simulink model "TosAeb"  (R2021a)
settings: Solver=FixedStepDiscrete  StartTime=0.0  StopTime=inf  FixedStep=0.01  RelTol=1e-3
undefined symbols (not defined in the file or in its folder): ACCModSts, AccSts1, AEB_ObjectMovingOrientation, AEB_ObjectMovingState, CN_BTCActive, CN_CurrentGearR, CN_CurrentGearR_DHT, CN_PTCActive, CN_VDCActive, CN_VSC_EspOff, CN_VSC_FCW_AEB_Error, CN_VSC_FCW_AEB_NoDispay, CN_VSC_FCW_AEB_PerfmDgdtn, CN_VSC_FCW_AEB_TempUnAvlbl, CN_VSC_VehStandstill, CNaccelAvoidMax, CNaccelAvoidMin, CNbrakePedalPress, CNcomfortStrLeftAccelMax, CNcomfortStrLeftAccelMin, CNcomfortStrRightAccelMax, CNcomfortStrRightAccelMin, CNDrvDoorOpen, CNDrvModeEco, CNDrvModeNorm, CNDrvModeOther, CNDrvModeSport, CNDrvSBRreminder, CNNull, CNobjInvalidID, CNPassDoorOpen, CNPassSBRreminder, CNPEBSAccelNegInf, CNPEBSAccelNull, CNPEBSTimeInf, CNPEBSTimeNull, CNSTOPMAX, CNSTOPNULL, CNsubjWidth, CNSwtOFF, CNSwtON, CNTTCMAX, CNTTCNULL, CNVehConfigAEBCycNone, CNVehConfigAEBNone, CNVehConfigAEBPedNone, CNVehConfigFCWNone, CNVehConfigJANone, CollisionSeverity, FALSE, FCWSnvty, JACycObjCalcInfoDefaultParams, JAPedObjCalcInfoDefaultParams, JAVehObjCalcInfoDefaultParams, latCycObjCalcInfoDefaultParams, latPedObjCalcInfoDefaultParams, longObjCalcInfoDefaultParams, ModSts, movingStatus, ObjectType ... (+1112)
libraries: simulink x3031, AEBSFunctionLib x50, Ta_Library x33, AEBSStateMachineLib x23, Mylibrary x12, simulink_extras x7, ObjSelect_lib x3, dspstat3 x2
(part 101 of 102)

== stateflow chart TosAeb/ELK_Objsel/Target_Selection/If Action Subsystem/ELK_TargetSelection/If Action Threat_Assessment_Left/Trigger Hold1/Chart ==
  (default) -> (junction 1)
  (junction 1) -> (junction 2) [order 1] : [(Trigger==1)]...
      | {count = 0;}
  (junction 1) -> (junction 12) [order 2]
  (junction 2) -> (junction 10)
  (junction 12) -> (junction 10) [order 1] : [(count<= hold_time)&&(count>0)]
  (junction 12) -> (junction 3) [order 2] : {Out = 0;}
  (junction 10) -> (junction 15) : {
      | Out = 1;...
      | count = count + 10;
      | }
  (junction 3) -> (junction 15)
  (junction 15) -> (junction 8)
  data: Trigger input; hold_time input; Out output double; count local double

== stateflow chart TosAeb/ELK_Objsel/Target_Selection/If Action Subsystem/ELK_TargetSelection/If Action Threat_Assessment_Left1/Delay_Trig/Chart ==
  state OFF
    en: Delay_Trig =0;
    count= 0;
    settings: description=_GWMH9P4.LKAFN.111_17_
  state ON
    en: Delay_Trig = 0;
    count++;
    du:count++;
    settings: description=_GWMH9P4.LKAFN.111_18_
  state Trig
    en: Delay_Trig = 1;
    settings: description=_GWMH9P4.LKAFN.111_19_
  (default) -> OFF
  OFF -> ON : [(Input)]
  ON -> OFF [order 2] : [!Input]
  Trig -> OFF : [!Input]
  ON -> Trig [order 1] : [(Input) && (count>= Delay_Trig_count)]
  data: Input input; Delay_Trig_count input; Delay_Trig output double; count local double

== stateflow chart TosAeb/ELK_Objsel/Target_Selection/If Action Subsystem/ELK_TargetSelection/If Action Threat_Assessment_Left1/Delay_Trig1/Chart ==
  state OFF
    en: Delay_Trig =0;
    count= 0;
    settings: description=_GWMH9P4.LKAFN.111_17_
  state ON
    en: Delay_Trig = 0;
    count++;
    du:count++;
    settings: description=_GWMH9P4.LKAFN.111_18_
  state Trig
    en: Delay_Trig = 1;
    settings: description=_GWMH9P4.LKAFN.111_19_
  (default) -> OFF
  OFF -> ON : [(Input)]
  ON -> OFF [order 2] : [!Input]
  Trig -> OFF : [!Input]
  ON -> Trig [order 1] : [(Input) && (count>= Delay_Trig_count)]
  data: Input input; Delay_Trig_count input; Delay_Trig output double; count local double

== stateflow chart TosAeb/ELK_Objsel/Target_Selection/If Action Subsystem/ELK_TargetSelection/If Action Threat_Assessment_Left1/Hysteresis ==
  state Flag_False
    en:
    Flag = 0;
    du:
    Flag = 0;
    settings: description=_GWMH9P5.ELKFN.111_3_
  state Flag_True
    en:
    Flag = 1;
    du:
    Flag = 1;
    settings: description=_GWMH9P5.ELKFN.111_4_
  (default) -> Flag_False
  Flag_False -> Flag_True : [Input_1 == 1]
  Flag_True -> Flag_False : [Input_2 == 1]
  data: Input_1 input; Input_2 input; Flag output double

== stateflow chart TosAeb/ELK_Objsel/Target_Selection/If Action Subsystem/ELK_TargetSelection/If Action Threat_Assessment_Left1/Hysteresis1 ==
  state Flag_False
    en:
    Flag = 0;
    du:
    Flag = 0;
    settings: description=_GWMH9P5.ELKFN.111_3_
  state Flag_True
    en:
    Flag = 1;
    du:
    Flag = 1;
    settings: description=_GWMH9P5.ELKFN.111_4_
  (default) -> Flag_False
  Flag_False -> Flag_True : [Input_1 == 1]
  Flag_True -> Flag_False : [Input_2 == 1]
  data: Input_1 input; Input_2 input; Flag output double

== stateflow chart TosAeb/ELK_Objsel/Target_Selection/If Action Subsystem/ELK_TargetSelection/If Action Threat_Assessment_Left1/Hysteresis2 ==
  state Flag_False
    en:
    Flag = 0;
    du:
    Flag = 0;
    settings: description=_GWMH9P5.ELKFN.111_3_
  state Flag_True
    en:
    Flag = 1;
    du:
    Flag = 1;
    settings: description=_GWMH9P5.ELKFN.111_4_
  (default) -> Flag_False
  Flag_False -> Flag_True : [Input_1 == 1]
  Flag_True -> Flag_False : [Input_2 == 1]
  data: Input_1 input; Input_2 input; Flag output double

== stateflow chart TosAeb/ELK_Objsel/Target_Selection/If Action Subsystem/ELK_TargetSelection/If Action Threat_Assessment_Left1/Trigger Hold/Chart ==
  (default) -> (junction 1)
  (junction 1) -> (junction 2) [order 1] : [(Trigger==1)]...
      | {count = 0;}
  (junction 1) -> (junction 12) [order 2]
  (junction 2) -> (junction 10)
  (junction 12) -> (junction 10) [order 1] : [(count<= hold_time)&&(count>0)]
  (junction 12) -> (junction 3) [order 2] : {Out = 0;}
  (junction 10) -> (junction 15) : {
      | Out = 1;...
      | count = count + 10;
      | }
  (junction 3) -> (junction 15)
  (junction 15) -> (junction 8)
  data: Trigger input; hold_time input; Out output double; count local double

== stateflow chart TosAeb/FcwAebJa_Step_sys/ALG_AEBSystem/ALG_AEBController/AEB_LatObjInfoCalcApp/LatPedObjCalc/Subsystem/latPedObjInfoCalc/ObjColiisionRiskProbCalc/CollisionRiskProbCalc/CollisionRiskProbCalc/dyOverlapFlow ==
  (default) -> (junction 32) : {
      | 	dyOverlapLimit = dyReginObjLLCorr- dyReginObjRUCorr;
      | 	dyOverlapLimit = max(dyOverlapLimit,0.1);
      | }
  (junction 32) -> (junction 34) [order 1] : [dyReginObjLLCorr < dyReginEgoRUCorr || ...
      | dyReginObjRUCorr > dyReginEgoLLCorr]
  (junction 32) -> (junction 2) [order 2]
  (junction 34) -> (junction 36) : {
      | 	dyOverlap = 0;
      | }
  (junction 2) -> (junction 3) [order 1] : [dyReginEgoLLCorr - dyReginEgoRUCorr < dyOverlapLimit && ...
      | dyReginEgoLLCorr <= dyReginObjLLCorr &&...
      | dyReginEgoRUCorr >= dyReginObjRUCorr]
  (junction 2) -> (junction 7) [order 2]
  (junction 3) -> (junction 5) : {
      | 	dyOverlap = dyReginEgoLLCorr - dyReginEgoRUCorr;
      | }
  (junction 7) -> (junction 9) [order 1] : [dyReginObjLLCorr - dyReginEgoRUCorr < dyOverlapLimit &&...
      | dyReginObjLLCorr - dyReginEgoRUCorr >0 && ...
      | dyReginEgoLLCorr > dyReginObjLLCorr]
  (junction 7) -> (junction 13) [order 2]
  (junction 9) -> (junction 11) : {
      | 	dyOverlap = dyReginObjLLCorr - dyReginEgoRUCorr;
      | }
  (junction 13) -> (junction 15) [order 1] : [dyReginEgoLLCorr - dyReginObjRUCorr < dyOverlapLimit && ...
      | dyReginEgoLLCorr - dyReginObjRUCorr > 0 && ...
      | dyReginEgoRUCorr < dyReginObjRUCorr]
  (junction 13) -> (junction 19) [order 2] : {
      | 	dyOverlap= dyOverlapLimit;
      | }
  (junction 15) -> (junction 17) : {
      | 	dyOverlap = dyReginEgoLLCorr - dyReginObjRUCorr;
      | }
  (junction 17) -> (junction 19)
  (junction 11) -> (junction 17)
  (junction 5) -> (junction 11)
  (junction 36) -> (junction 5)
  (junction 19) -> (junction 22)
  data: dyReginEgoRUCorr input single; dyReginEgoLLCorr input single; dyReginObjRUCorr input single; dyReginObjLLCorr input single; dyOverlap output single; dyOverlapLimit output single

== stateflow chart TosAeb/ELK_Objsel/Target_Selection/If Action Subsystem/ELK_TargetSelection/If Action Threat_Assessment_Left1/Trigger Hold1/Chart ==
  (default) -> (junction 1)
  (junction 1) -> (junction 2) [order 1] : [(Trigger==1)]...
      | {count = 0;}
  (junction 1) -> (junction 12) [order 2]
  (junction 2) -> (junction 10)
  (junction 12) -> (junction 10) [order 1] : [(count<= hold_time)&&(count>0)]
  (junction 12) -> (junction 3) [order 2] : {Out = 0;}
  (junction 10) -> (junction 15) : {
      | Out = 1;...
      | count = count + 10;
      | }
  (junction 3) -> (junction 15)
  (junction 15) -> (junction 8)
  data: Trigger input; hold_time input; Out output double; count local double

== stateflow chart TosAeb/ELK_Objsel/Target_Selection/If Action Subsystem/ELK_TargetSelection/If Action Threat_Assessment_Right/Delay_Trig/Chart ==
  state OFF
    en: Delay_Trig =0;
    count= 0;
    settings: description=_GWMH9P4.LKAFN.111_17_
  state ON
    en: Delay_Trig = 0;
    count++;
    du:count++;
    settings: description=_GWMH9P4.LKAFN.111_18_
  state Trig
    en: Delay_Trig = 1;
    settings: description=_GWMH9P4.LKAFN.111_19_
  (default) -> OFF
  OFF -> ON : [(Input)]
  ON -> OFF [order 2] : [!Input]
  Trig -> OFF : [!Input]
  ON -> Trig [order 1] : [(Input) && (count>= Delay_Trig_count)]
  data: Input input; Delay_Trig_count input; Delay_Trig output double; count local double

== stateflow chart TosAeb/ELK_Objsel/Target_Selection/If Action Subsystem/ELK_TargetSelection/If Action Threat_Assessment_Right/Delay_Trig1/Chart ==
  state OFF
    en: Delay_Trig =0;
    count= 0;
    settings: description=_GWMH9P4.LKAFN.111_17_
  state ON
    en: Delay_Trig = 0;
    count++;
    du:count++;
    settings: description=_GWMH9P4.LKAFN.111_18_
  state Trig
    en: Delay_Trig = 1;
    settings: description=_GWMH9P4.LKAFN.111_19_
  (default) -> OFF
  OFF -> ON : [(Input)]
  ON -> OFF [order 2] : [!Input]
  Trig -> OFF : [!Input]
  ON -> Trig [order 1] : [(Input) && (count>= Delay_Trig_count)]
  data: Input input; Delay_Trig_count input; Delay_Trig output double; count local double

== stateflow chart TosAeb/ELK_Objsel/Target_Selection/If Action Subsystem/ELK_TargetSelection/If Action Threat_Assessment_Right/Hysteresis ==
  state Flag_False
    en:
    Flag = 0;
    du:
    Flag = 0;
    settings: description=_GWMH9P5.ELKFN.111_3_
  state Flag_True
    en:
    Flag = 1;
    du:
    Flag = 1;
    settings: description=_GWMH9P5.ELKFN.111_4_
  (default) -> Flag_False
  Flag_False -> Flag_True : [Input_1 == 1]
  Flag_True -> Flag_False : [Input_2 == 1]
  data: Input_1 input; Input_2 input; Flag output double

== stateflow chart TosAeb/ELK_Objsel/Target_Selection/If Action Subsystem/ELK_TargetSelection/If Action Threat_Assessment_Right/Hysteresis1 ==
  state Flag_False
    en:
    Flag = 0;
    du:
    Flag = 0;
    settings: description=_GWMH9P5.ELKFN.111_3_
  state Flag_True
    en:
    Flag = 1;
    du:
    Flag = 1;
    settings: description=_GWMH9P5.ELKFN.111_4_
  (default) -> Flag_False
  Flag_False -> Flag_True : [Input_1 == 1]
  Flag_True -> Flag_False : [Input_2 == 1]
  data: Input_1 input; Input_2 input; Flag output double

== stateflow chart TosAeb/ELK_Objsel/Target_Selection/If Action Subsystem/ELK_TargetSelection/If Action Threat_Assessment_Right/Hysteresis2 ==
  state Flag_False
    en:
    Flag = 0;
    du:
    Flag = 0;
    settings: description=_GWMH9P5.ELKFN.111_3_
  state Flag_True
    en:
    Flag = 1;
    du:
    Flag = 1;
    settings: description=_GWMH9P5.ELKFN.111_4_
  (default) -> Flag_False
  Flag_False -> Flag_True : [Input_1 == 1]
  Flag_True -> Flag_False : [Input_2 == 1]
  data: Input_1 input; Input_2 input; Flag output double

== stateflow chart TosAeb/ELK_Objsel/Target_Selection/If Action Subsystem/ELK_TargetSelection/If Action Threat_Assessment_Right/Trigger Hold/Chart ==
  (default) -> (junction 1)
  (junction 1) -> (junction 2) [order 1] : [(Trigger==1)]...
      | {count = 0;}
  (junction 1) -> (junction 12) [order 2]
  (junction 2) -> (junction 10)
  (junction 12) -> (junction 10) [order 1] : [(count<= hold_time)&&(count>0)]
  (junction 12) -> (junction 3) [order 2] : {Out = 0;}
  (junction 10) -> (junction 15) : {
      | Out = 1;...
      | count = count + 10;
      | }
  (junction 3) -> (junction 15)
  (junction 15) -> (junction 8)
  data: Trigger input; hold_time input; Out output double; count local double

== stateflow chart TosAeb/ELK_Objsel/Target_Selection/If Action Subsystem/ELK_TargetSelection/If Action Threat_Assessment_Right/Trigger Hold1/Chart ==
  (default) -> (junction 1)
  (junction 1) -> (junction 2) [order 1] : [(Trigger==1)]...
      | {count = 0;}
  (junction 1) -> (junction 12) [order 2]
  (junction 2) -> (junction 10)
  (junction 12) -> (junction 10) [order 1] : [(count<= hold_time)&&(count>0)]
  (junction 12) -> (junction 3) [order 2] : {Out = 0;}
  (junction 10) -> (junction 15) : {
      | Out = 1;...
      | count = count + 10;
      | }
  (junction 3) -> (junction 15)
  (junction 15) -> (junction 8)
  data: Trigger input; hold_time input; Out output double; count local double

== stateflow chart TosAeb/ELK_Objsel/Target_Selection/If Action Subsystem/ELK_TargetSelection/If Action Threat_Assessment_Right1/Delay_Trig/Chart ==
  state OFF
    en: Delay_Trig =0;
    count= 0;
    settings: description=_GWMH9P4.LKAFN.111_17_
  state ON
    en: Delay_Trig = 0;
    count++;
    du:count++;
    settings: description=_GWMH9P4.LKAFN.111_18_
  state Trig
    en: Delay_Trig = 1;
    settings: description=_GWMH9P4.LKAFN.111_19_
  (default) -> OFF
  OFF -> ON : [(Input)]
  ON -> OFF [order 2] : [!Input]
  Trig -> OFF : [!Input]
  ON -> Trig [order 1] : [(Input) && (count>= Delay_Trig_count)]
  data: Input input; Delay_Trig_count input; Delay_Trig output double; count local double

== stateflow chart TosAeb/ELK_Objsel/Target_Selection/If Action Subsystem/ELK_TargetSelection/If Action Threat_Assessment_Right1/Delay_Trig1/Chart ==
  state OFF
    en: Delay_Trig =0;
    count= 0;
    settings: description=_GWMH9P4.LKAFN.111_17_
  state ON
    en: Delay_Trig = 0;
    count++;
    du:count++;
    settings: description=_GWMH9P4.LKAFN.111_18_
  state Trig
    en: Delay_Trig = 1;
    settings: description=_GWMH9P4.LKAFN.111_19_
  (default) -> OFF
  OFF -> ON : [(Input)]
  ON -> OFF [order 2] : [!Input]
  Trig -> OFF : [!Input]
  ON -> Trig [order 1] : [(Input) && (count>= Delay_Trig_count)]
  data: Input input; Delay_Trig_count input; Delay_Trig output double; count local double

== stateflow chart TosAeb/ELK_Objsel/Target_Selection/If Action Subsystem/ELK_TargetSelection/If Action Threat_Assessment_Right1/Hysteresis ==
  state Flag_False
    en:
    Flag = 0;
    du:
    Flag = 0;
    settings: description=_GWMH9P5.ELKFN.111_3_
  state Flag_True
    en:
    Flag = 1;
    du:
    Flag = 1;
    settings: description=_GWMH9P5.ELKFN.111_4_
  (default) -> Flag_False
  Flag_False -> Flag_True : [Input_1 == 1]
  Flag_True -> Flag_False : [Input_2 == 1]
  data: Input_1 input; Input_2 input; Flag output double

== stateflow chart TosAeb/ELK_Objsel/Target_Selection/If Action Subsystem/ELK_TargetSelection/If Action Threat_Assessment_Right1/Hysteresis1 ==
  state Flag_False
    en:
    Flag = 0;
    du:
    Flag = 0;
    settings: description=_GWMH9P5.ELKFN.111_3_
  state Flag_True
    en:
    Flag = 1;
    du:
    Flag = 1;
    settings: description=_GWMH9P5.ELKFN.111_4_
  (default) -> Flag_False
  Flag_False -> Flag_True : [Input_1 == 1]
  Flag_True -> Flag_False : [Input_2 == 1]
  data: Input_1 input; Input_2 input; Flag output double

== stateflow chart TosAeb/ELK_Objsel/Target_Selection/If Action Subsystem/ELK_TargetSelection/If Action Threat_Assessment_Right1/Hysteresis2 ==
  state Flag_False
    en:
    Flag = 0;
    du:
    Flag = 0;
    settings: description=_GWMH9P5.ELKFN.111_3_
  state Flag_True
    en:
    Flag = 1;
    du:
    Flag = 1;
    settings: description=_GWMH9P5.ELKFN.111_4_
  (default) -> Flag_False
  Flag_False -> Flag_True : [Input_1 == 1]
  Flag_True -> Flag_False : [Input_2 == 1]
  data: Input_1 input; Input_2 input; Flag output double

== stateflow chart TosAeb/ELK_Objsel/Target_Selection/If Action Subsystem/ELK_TargetSelection/If Action Threat_Assessment_Right1/Trigger Hold/Chart ==
  (default) -> (junction 1)
  (junction 1) -> (junction 2) [order 1] : [(Trigger==1)]...
      | {count = 0;}
  (junction 1) -> (junction 12) [order 2]
  (junction 2) -> (junction 10)
  (junction 12) -> (junction 10) [order 1] : [(count<= hold_time)&&(count>0)]
  (junction 12) -> (junction 3) [order 2] : {Out = 0;}
  (junction 10) -> (junction 15) : {
      | Out = 1;...
      | count = count + 10;
      | }
  (junction 3) -> (junction 15)
  (junction 15) -> (junction 8)
  data: Trigger input; hold_time input; Out output double; count local double

== stateflow chart TosAeb/ELK_Objsel/Target_Selection/If Action Subsystem/ELK_TargetSelection/If Action Threat_Assessment_Right1/Trigger Hold1/Chart ==
  (default) -> (junction 1)
  (junction 1) -> (junction 2) [order 1] : [(Trigger==1)]...
      | {count = 0;}
  (junction 1) -> (junction 12) [order 2]
  (junction 2) -> (junction 10)
  (junction 12) -> (junction 10) [order 1] : [(count<= hold_time)&&(count>0)]
  (junction 12) -> (junction 3) [order 2] : {Out = 0;}
  (junction 10) -> (junction 15) : {
      | Out = 1;...
      | count = count + 10;
      | }
  (junction 3) -> (junction 15)
  (junction 15) -> (junction 8)
  data: Trigger input; hold_time input; Out output double; count local double

== matlab function TosAeb/ELK_Objsel/Target_Selection/If Action Subsystem/MATLAB Function ==
  data: dxv_f input; vxvAbs_f output; dyv_f input; vxvRel_f input; vyvRel_f input; vxvEgo_f input; psiDtEgo_f input; vyvAbs_f output; vrAbs_f output
function [vxvAbs_f, vyvAbs_f, vrAbs_f] = fcn(dxv_f, dyv_f, vxvRel_f, vyvRel_f, vxvEgo_f, psiDtEgo_f)

if (dxv_f ~= 0)
   t_radObjAngleToRear_f = atan(dyv_f / dxv_f);
else
   t_radObjAngleToRear_f = single(0.0);
end

t_dvRadial_f = sqrt(dxv_f * dxv_f + dyv_f * dyv_f);

t_vvRadial_f = t_dvRadial_f * psiDtEgo_f;

t_sinObjAngle_f = sin(t_radObjAngleToRear_f);
t_cosObjAngle_f = cos(t_radObjAngleToRear_f);

t_tempvx_f = (vxvRel_f * t_cosObjAngle_f) + vyvRel_f * t_sinObjAngle_f;
t_tempvy_f = (vxvRel_f * (-t_sinObjAngle_f)) + vyvRel_f * t_cosObjAngle_f;

t_tempvy_f = t_tempvy_f + t_vvRadial_f;
if dxv_f <single(0)
    vxvAbs_f = (-1)*((t_tempvx_f * t_cosObjAngle_f) - (t_tempvy_f * t_sinObjAngle_f)) + vxvEgo_f;
else
    vxvAbs_f = ((t_tempvx_f * t_cosObjAngle_f) - (t_tempvy_f * t_sinObjAngle_f)) + vxvEgo_f;
end
vyvAbs_f = (t_tempvx_f * t_sinObjAngle_f) + (t_tempvy_f * t_cosObjAngle_f);
vrAbs_f = sqrt(vxvAbs_f * vxvAbs_f + vyvAbs_f * vyvAbs_f);

== matlab function TosAeb/ELK_Objsel/Target_Selection/Subsystem/state_rebuild/State_match ==
  data: state input; obj_state output; orien input; vy input
function obj_state = fcn(state,orien,vy)
if state == uint8(1) && orien == uint8(0)
    obj_state = uint8(1);
elseif state == uint8(2)
    obj_state = uint8(3);
elseif state == uint8(3)&&(orien ~= uint8(0))
    obj_state = uint8(5);
    if orien == uint8(1)
        obj_state = uint8(5);
    elseif orien == uint8(2)
        obj_state = uint8(8);
    elseif orien == uint8(3)
        if vy > single(0)
            obj_state = uint8(10);
        elseif vy<single(0)
            obj_state = uint8(11);
        else
            obj_state = uint8(9);
        end
    end
else
    obj_state = uint8(0);
end

== matlab function TosAeb/ELK_Objsel/Target_Selection/Subsystem/type_rebuild/type_match ==
  data: type input; type_re output
function type_re = fcn(type)
if (type >= uint8(0) && type <= uint8(3))||(type >= uint8(16) && type <= uint8(18)) %car/suv loory/minivan/4 wheeler/gerneral small vehicle/gerneral large vehicle
    type_re = uint8(3);
elseif type == uint8(4) % bus
    type_re = uint8(5);
elseif type == uint8(5) %truck
    type_re = uint8(5);
elseif type == uint8(6) %pedestrian
    type_re = uint8(1);
elseif type == uint8(7) %trucycle
    type_re = uint8(6);
elseif type == uint8(8) %bicycle
    type_re = uint8(17);
elseif type == uint8(9) %motor
    type_re = uint8(2);
elseif type == uint8(10) %traffic cone  锥形桶
    type_re = uint8(10);
elseif type == uint8(11) ||type == uint8(14)  %barrier
    type_re = uint8(23);
elseif type == uint8(12) %traffic light
    type_re = uint8(13);  %warning post
elseif type == uint8(13)
    type_re = uint8(0);
elseif type == uint8(15)  %general 2 wheeler
    type_re = uint8(17);
else
    type_re = uint8(0);
end

end

== stateflow chart TosAeb/FcwAebJa_Step_sys/ALG_AEBSystem/ALG_AEBOutput/AEBS_BrkInfoCalc/AEB_BrkReqFuncCalc/Inter_AEB_BrkReqFuncFlow ==
  (default) -> (junction 2)
  (junction 2) -> (junction 3) [order 1] : [AEBfullFuncImpArb == ModSts.ActiveState ||...
      | AEBpartFuncImpArb == ModSts.ActiveState ||...
      | EBAFuncImpArb == ModSts.ActiveState]
  (junction 2) -> (junction 17) [order 2]
  (junction 3) -> (junction 5) : {
      | 	temp = 1;
      | }
  (junction 17) -> (junction 19) [order 1] : [pedAEBfullFuncImpArb == ModSts.ActiveState ||...
      | cycAEBpartFuncImpArb == ModSts.ActiveState ||...
      | cycAEBfullFuncImpArb == ModSts.ActiveState ]
  (junction 17) -> (junction 23) [order 2]
  (junction 19) -> (junction 21) : {
      | 	temp = 2;
      | }
  (junction 23) -> (junction 25) [order 1] : [JA_AEBfullFuncImpArb == ModSts.ActiveState ||...
      | JA_pedAEBRawModSts == ModSts.ActiveState]
  (junction 23) -> (junction 29) [order 2]
  (junction 25) -> (junction 27) : {
      | 	temp = 3;
      | }
  (junction 29) -> (junction 32) [order 1] : [FCTBFuncImpArb == ModSts.ActiveState]
  (junction 29) -> (junction 36) [order 2] : {
      | 	temp = 0;
      | }
  (junction 32) -> (junction 34) : {
      | 	temp = 5;
      | }
  (junction 34) -> (junction 36)
  (junction 27) -> (junction 34)
  (junction 21) -> (junction 27)
  (junction 5) -> (junction 21)
  (junction 36) -> (junction 40) : {
      | 	AEB_BrkReqFunc = temp;
      | }
  data: AEBpartFuncImpArb input; AEBfullFuncImpArb input; EBAFuncImpArb input; cycAEBpartFuncImpArb input; cycAEBfullFuncImpArb input; pedAEBfullFuncImpArb input; JA_AEBfullFuncImpArb input; JA_pedAEBRawModSts input; FCTBFuncImpArb input; AEB_BrkReqFunc output uint8; temp local uint8

== stateflow chart TosAeb/FcwAebJa_Step_sys/ALG_AEBSystem/ALG_AEBOutput/AEBS_DVRInfoCalc/AEB_JAPED_DVR_INFO/Subsystem/AEBObjStateMap4/AEBObjStateFlow ==
  (default) -> (junction 2)
  (junction 2) -> (junction 3) [order 1] : [ObjectMovingState == AEB_ObjectMovingState.Moving &&...
      |  (ObjectMovingOrientation == AEB_ObjectMovingOrientation.SameDir||...
      | ObjectMovingOrientation == AEB_ObjectMovingOrientation.Crossing)]
  (junction 2) -> (junction 7) [order 2]
  (junction 3) -> (junction 5) : {
      | AEBObjState = uint8(1);
      | }
  (junction 7) -> (junction 9) [order 1] : [ObjectMovingState == AEB_ObjectMovingState.Still]
  (junction 7) -> (junction 13) [order 2]
  (junction 9) -> (junction 11) : {
      | AEBObjState = uint8(2);
      | }
  (junction 13) -> (junction 15) [order 1] : [ObjectMovingState == AEB_ObjectMovingState.Stopped]
  (junction 13) -> (junction 19) [order 2]
  (junction 15) -> (junction 17) : {AEBObjState = uint8(3);}
  (junction 19) -> (junction 21) [order 1] : [ObjectMovingState == AEB_ObjectMovingOrientation.InvDir]
  (junction 19) -> (junction 26) [order 2] : {AEBObjState = uint8(0);}
  (junction 21) -> (junction 24) : {AEBObjState = uint8(3);}
  (junction 24) -> (junction 26)
  (junction 17) -> (junction 24)
  (junction 11) -> (junction 17)
  (junction 5) -> (junction 11)
  (junction 26) -> (junction 32)
  data: ObjectMovingState input Enum: AEB_ObjectMovingState; ObjectMovingOrientation input Enum: AEB_ObjectMovingOrientation; AEBObjState output uint8

== stateflow chart TosAeb/FcwAebJa_Step_sys/ALG_AEBSystem/ALG_AEBOutput/AEBS_DVRInfoCalc/AEB_JAVEH_DVR_INFO/Subsystem/AEBObjStateMap/AEBObjStateFlow ==
  (default) -> (junction 2)
  (junction 2) -> (junction 3) [order 1] : [ObjectMovingState == AEB_ObjectMovingState.Moving &&...
      |  (ObjectMovingOrientation == AEB_ObjectMovingOrientation.SameDir||...
      | ObjectMovingOrientation == AEB_ObjectMovingOrientation.Crossing)]
  (junction 2) -> (junction 7) [order 2]
  (junction 3) -> (junction 5) : {
      | AEBObjState = uint8(1);
      | }
  (junction 7) -> (junction 9) [order 1] : [ObjectMovingState == AEB_ObjectMovingState.Still]
  (junction 7) -> (junction 13) [order 2]
  (junction 9) -> (junction 11) : {
      | AEBObjState = uint8(2);
      | }
  (junction 13) -> (junction 15) [order 1] : [ObjectMovingState == AEB_ObjectMovingState.Stopped]
  (junction 13) -> (junction 19) [order 2]
  (junction 15) -> (junction 17) : {AEBObjState = uint8(3);}
  (junction 19) -> (junction 21) [order 1] : [ObjectMovingState == AEB_ObjectMovingOrientation.InvDir]
  (junction 19) -> (junction 26) [order 2] : {AEBObjState = uint8(0);}
  (junction 21) -> (junction 24) : {AEBObjState = uint8(3);}
  (junction 24) -> (junction 26)
  (junction 17) -> (junction 24)
  (junction 11) -> (junction 17)
  (junction 5) -> (junction 11)
  (junction 26) -> (junction 32)
  data: ObjectMovingState input Enum: AEB_ObjectMovingState; ObjectMovingOrientation input Enum: AEB_ObjectMovingOrientation; AEBObjState output uint8

== stateflow chart TosAeb/FcwAebJa_Step_sys/ALG_AEBSystem/ALG_AEBOutput/AEBS_DVRInfoCalc/AEB_LATCYC_DVR_INFO/Subsystem/AEBObjStateMap/AEBObjStateFlow ==
  (default) -> (junction 2)
  (junction 2) -> (junction 3) [order 1] : [ObjectMovingState == AEB_ObjectMovingState.Moving &&...
      |  (ObjectMovingOrientation == AEB_ObjectMovingOrientation.SameDir||...
      | ObjectMovingOrientation == AEB_ObjectMovingOrientation.Crossing)]
  (junction 2) -> (junction 7) [order 2]
  (junction 3) -> (junction 5) : {
      | AEBObjState = uint8(1);
      | }
  (junction 7) -> (junction 9) [order 1] : [ObjectMovingState == AEB_ObjectMovingState.Still]
  (junction 7) -> (junction 13) [order 2]
  (junction 9) -> (junction 11) : {
      | AEBObjState = uint8(2);
      | }
  (junction 13) -> (junction 15) [order 1] : [ObjectMovingState == AEB_ObjectMovingState.Stopped]
  (junction 13) -> (junction 19) [order 2]
  (junction 15) -> (junction 17) : {AEBObjState = uint8(3);}
  (junction 19) -> (junction 21) [order 1] : [ObjectMovingState == AEB_ObjectMovingOrientation.InvDir]
  (junction 19) -> (junction 26) [order 2] : {AEBObjState = uint8(0);}
  (junction 21) -> (junction 24) : {AEBObjState = uint8(3);}
  (junction 24) -> (junction 26)
  (junction 17) -> (junction 24)
  (junction 11) -> (junction 17)
  (junction 5) -> (junction 11)
  (junction 26) -> (junction 32)
  data: ObjectMovingState input Enum: AEB_ObjectMovingState; ObjectMovingOrientation input Enum: AEB_ObjectMovingOrientation; AEBObjState output uint8

== stateflow chart TosAeb/FcwAebJa_Step_sys/ALG_AEBSystem/ALG_AEBController/AEB_FuncImpManagementApp/FuncManeuArbCalc/brkFuncManeuArbCalc/axTgtReqValueCalc1/AebfullTriggerInfoCalc/AEBTriggerRelCalc/AEBTriggerInfo/AEBTriggerInfo ==
  state AEB_Standby
    en:
    FuncActvationTimeAtStart = single(0);
    vxPredictedAtStart = single(0);
    %vxAtStart = 0;
    subjVelAtStart = single(0);
    dxSecureAtStart = single(0);
    AebActiveFirstCycle = false;
    AebFullFuncStatus = ModSts.StandbyState
  state dxSecure = dxSecureCalc(subjVel,vxPredicted) [function]
  state AEB_Active
    en:
    FuncActvationTimeAtStart = FuncActvationTime;
    vxPredictedAtStart = vxPredicted;
    %vxAtStart = vx;
    subjVelAtStart = subjVel;
    dxSecureAtStart = dxSecureCalc(subjVel,vxPredicted);
    AebActiveFirstCycle = true;
    AebFullFuncStatus = ModSts.ActiveState
    du:
    AebActiveFirstCycle = false;
  state AEB_StandstillActive
    en:
    AebFullFuncStatus = ModSts.StandstillActiveState
  (default) -> AEB_Standby
  (junction 25) -> AEB_Standby
  (junction 23) -> (junction 25) : [AebActive == false]
  AEB_Active -> AEB_Standby [order 1] : [AebActive == false]
  AEB_Standby -> AEB_Active : [AebActive == true]
  AEB_Active -> AEB_StandstillActive [order 2] : [AebActive == true &&...
      | VSC_VehStandstill == true]
  AEB_StandstillActive -> (junction 23)
  data: subjVel function_input; vxPredicted function_input; dxSecure function_output; AebFullFuncStatus output Enum: ModSts; AebActiveFirstCycle output boolean; AebActive input; FuncActvationTime input; subjVel input; vxPredicted input; FuncActvationTimeAtStart output single; dxSecureAtStart output single; subjVelAtStart output single; vxPredictedAtStart output single; VSC_VehStandstill input

== stateflow chart TosAeb/FcwAebJa_Step_sys/ALG_AEBSystem/ALG_AEBOutput/AEBS_DVRInfoCalc/AEB_LATPED_DVR_INFO/Subsystem/AEBObjStateMap/AEBObjStateFlow ==
  (default) -> (junction 2)
  (junction 2) -> (junction 3) [order 1] : [ObjectMovingState == AEB_ObjectMovingState.Moving &&...
      |  (ObjectMovingOrientation == AEB_ObjectMovingOrientation.SameDir||...
      | ObjectMovingOrientation == AEB_ObjectMovingOrientation.Crossing)]
  (junction 2) -> (junction 7) [order 2]
  (junction 3) -> (junction 5) : {
      | AEBObjState = uint8(1);
      | }
  (junction 7) -> (junction 9) [order 1] : [ObjectMovingState == AEB_ObjectMovingState.Still]
  (junction 7) -> (junction 13) [order 2]
  (junction 9) -> (junction 11) : {
      | AEBObjState = uint8(2);
      | }
  (junction 13) -> (junction 15) [order 1] : [ObjectMovingState == AEB_ObjectMovingState.Stopped]
  (junction 13) -> (junction 19) [order 2]
  (junction 15) -> (junction 17) : {AEBObjState = uint8(3);}
  (junction 19) -> (junction 21) [order 1] : [ObjectMovingState == AEB_ObjectMovingOrientation.InvDir]
  (junction 19) -> (junction 26) [order 2] : {AEBObjState = uint8(0);}
  (junction 21) -> (junction 24) : {AEBObjState = uint8(3);}
  (junction 24) -> (junction 26)
  (junction 17) -> (junction 24)
  (junction 11) -> (junction 17)
  (junction 5) -> (junction 11)
  (junction 26) -> (junction 32)
  data: ObjectMovingState input Enum: AEB_ObjectMovingState; ObjectMovingOrientation input Enum: AEB_ObjectMovingOrientation; AEBObjState output uint8

== stateflow chart TosAeb/FcwAebJa_Step_sys/ALG_AEBSystem/ALG_AEBOutput/AEBS_DVRInfoCalc/AEB_LON_DVR_INFO/If Action Subsystem/AEBObjStateMap/AEBObjStateFlow ==
  (default) -> (junction 2)
  (junction 2) -> (junction 3) [order 1] : [ObjectMovingState == AEB_ObjectMovingState.Moving &&...
      |  (ObjectMovingOrientation == AEB_ObjectMovingOrientation.SameDir||...
      | ObjectMovingOrientation == AEB_ObjectMovingOrientation.Crossing)]
  (junction 2) -> (junction 7) [order 2]
  (junction 3) -> (junction 5) : {
      | AEBObjState = uint8(1);
      | }
  (junction 7) -> (junction 9) [order 1] : [ObjectMovingState == AEB_ObjectMovingState.Still]
  (junction 7) -> (junction 13) [order 2]
  (junction 9) -> (junction 11) : {
      | AEBObjState = uint8(2);
      | }
  (junction 13) -> (junction 15) [order 1] : [ObjectMovingState == AEB_ObjectMovingState.Stopped]
  (junction 13) -> (junction 19) [order 2]
  (junction 15) -> (junction 17) : {AEBObjState = uint8(3);}
  (junction 19) -> (junction 21) [order 1] : [ObjectMovingState == AEB_ObjectMovingOrientation.InvDir]
  (junction 19) -> (junction 26) [order 2] : {AEBObjState = uint8(0);}
  (junction 21) -> (junction 24) : {AEBObjState = uint8(3);}
  (junction 24) -> (junction 26)
  (junction 17) -> (junction 24)
  (junction 11) -> (junction 17)
  (junction 5) -> (junction 11)
  (junction 26) -> (junction 32)
  data: ObjectMovingState input Enum: AEB_ObjectMovingState; ObjectMovingOrientation input Enum: AEB_ObjectMovingOrientation; AEBObjState output uint8

== stateflow chart TosAeb/FcwAebJa_Step_sys/ALG_AEBSystem/ALG_AEBOutput/AEBS_IPHUTInfoCalc/AEB_ObjStyMap/AEB_ObjStyFlow ==
  note: 0x0:No Object
      | 0x1:Vehicle
      | 0x2:Pedestrian
      | 0x3:Cyclist
      | 0x4:unknown
      | 0x5~0x7:Resvered
  state OutType = DadtaTypeConvert(type) [function]
    note: 0x0:No Object
        | 0x1:Vehicle
        | 0x2:Pedestrian
        | 0x3:Cyclist
        | 0x4:unknown
        | 0x5~0x7:Resvered
    (default) -> (junction 119)
    (junction 119) -> (junction 120) [order 1] : [type == ObjectType.CAR]
    (junction 119) -> (junction 123) [order 2]
    (junction 120) -> (junction 121) : {
        | 	OutType = uint8(1);
        | }
    (junction 121) -> (junction 122)
    (junction 122) -> (junction 126)
    (junction 123) -> (junction 124) [order 1] : [type == ObjectType.BICYCLE]
    (junction 123) -> (junction 127) [order 2]
    (junction 124) -> (junction 125) : {
        | 	OutType = uint8(3);
        | }
    (junction 125) -> (junction 126)
    (junction 126) -> (junction 130)
    (junction 127) -> (junction 128) [order 1] : [type == ObjectType.PEDESTRIAN]
    (junction 127) -> (junction 135) [order 2] : {
        | 	OutType = uint8(4);
        | }
    (junction 128) -> (junction 129) : {
        | 	OutType = uint8(2);
        | }
    (junction 129) -> (junction 130)
    (junction 130) -> (junction 136)
    (junction 136) -> (junction 135)
    (junction 135) -> (junction 137)
  (default) -> (junction 2)
  (junction 2) -> (junction 3) [order 1] : [AEBTrigger == TRUE]
  (junction 2) -> (junction 18) [order 2] : {
      | 	Inter_AEB_ObjSty = uint8(0);
      | }
  (junction 3) -> (junction 5)
  (junction 5) -> (junction 7) [order 1] : [EBAfullFuncImpArb == ModSts.ActiveState ||...
      | AEBfullFuncImpArb == ModSts.ActiveState ||...
      | AEBPartFuncImpArb == ModSts.ActiveState]
  (junction 5) -> (junction 12) [order 2]
  (junction 7) -> (junction 9) : {
      | 	temp_AEB_ObjSty = longObjectType;
      | }
  (junction 12) -> (junction 14) [order 1] : [pedAEBfullFuncImpArb == ModSts.ActiveState]
  (junction 12) -> (junction 20) [order 2]
  (junction 14) -> (junction 16) : {
      | 	temp_AEB_ObjSty = latPedObjectType;
      | }
  (junction 20) -> (junction 22) [order 1] : [cycAEBfullFuncImpArb == ModSts.ActiveState ||...
      | cycAEBpartFuncImpArb == ModSts.ActiveState]
  (junction 20) -> (junction 26) [order 2]
  (junction 22) -> (junction 24) : {
      | 	temp_AEB_ObjSty = latCycObjectType;
      | }
  (junction 26) -> (junction 28) [order 1] : [JA_AEBfullFuncImpArb == ModSts.ActiveState]
  (junction 26) -> (junction 32) [order 2]
  (junction 28) -> (junction 142) : {%此接口为接入JA目标类型
      | 	temp_AEB_ObjSty = JaVehObjectType;
      | }
  (junction 32) -> (junction 34) [order 1] : [JA_pedAEBfullFuncImpArb == ModSts.ActiveState]
  (junction 32) -> (junction 38) [order 2] : {
      | 	Inter_AEB_ObjSty = uint8(0);
      | }
  (junction 34) -> (junction 36) : {
      | 	temp_AEB_ObjSty = JaPedObjectType;
      | }
  (junction 142) -> (junction 36)
  (junction 24) -> (junction 142)
  (junction 16) -> (junction 24)
  (junction 9) -> (junction 16)
  (junction 36) -> (junction 75) : {
      | 	Inter_AEB_ObjSty = DadtaTypeConvert(temp_AEB_ObjSty);
      | }
  (junction 38) -> (junction 18)
  (junction 75) -> (junction 38)
  (junction 18) -> (junction 42)
  data: type function_input Enum: ObjectType size=1; OutType function_output uint8 size=1; AEBTrigger input boolean; AEBPartFuncImpArb input Enum: ModSts; EBAfullFuncImpArb input Enum: ModSts; AEBfullFuncImpArb input Enum: ModSts; pedAEBfullFuncImpArb input Enum: ModSts; cycAEBpartFuncImpArb input Enum: ModSts; cycAEBfullFuncImpArb input Enum: ModSts; JA_AEBfullFuncImpArb input Enum: ModSts; JA_pedAEBfullFuncImpArb input Enum: ModSts; TRUE parameter boolean; Inter_AEB_ObjSty output uint8; longObjectType input Enum: ObjectType; latPedObjectType input Enum: ObjectType; latCycObjectType input Enum: ObjectType; temp_AEB_ObjSty local Enum: ObjectType size=1 =ObjectType.CAR; JaPedObjectType input Enum: ObjectType; JaVehObjectType input Enum: ObjectType

== stateflow chart TosAeb/FcwAebJa_Step_sys/ALG_AEBSystem/ALG_AEBOutput/AEBS_IPHUTInfoCalc/AEB_ObjStyMap1/AEB_ObjStyFlow ==
  note: 0x0:No Object
      | 0x1:Vehicle
      | 0x2:Pedestrian
      | 0x3:Cyclist
      | 0x4:unknown
      | 0x5~0x7:Resvered
  (default) -> (junction 2)
  (junction 2) -> (junction 3) [order 1] : [AEBTrigger == TRUE]
  (junction 2) -> (junction 18) [order 2] : {
      | 	temp_AEB_ObjSty = uint8(0);
      | }
  (junction 3) -> (junction 5)
  (junction 5) -> (junction 7) [order 1] : [EBAfullFuncImpArb == ModSts.ActiveState ||...
      | AEBfullFuncImpArb == ModSts.ActiveState ||...
      | AEBPartFuncImpArb == ModSts.ActiveState]
  (junction 5) -> (junction 12) [order 2]
  (junction 7) -> (junction 9) : {
      | 	temp_AEB_ObjSty = uint8(1);
      | }
  (junction 12) -> (junction 14) [order 1] : [pedAEBfullFuncImpArb == ModSts.ActiveState]
  (junction 12) -> (junction 20) [order 2]
  (junction 14) -> (junction 16) : {
      | 	temp_AEB_ObjSty = uint8(2);
      | }
  (junction 20) -> (junction 22) [order 1] : [cycAEBfullFuncImpArb == ModSts.ActiveState ||...
      | cycAEBpartFuncImpArb == ModSts.ActiveState]
  (junction 20) -> (junction 26) [order 2]
  (junction 22) -> (junction 24) : {
      | 	temp_AEB_ObjSty = uint8(3);
      | }
  (junction 26) -> (junction 28) [order 1] : [JA_AEBfullFuncImpArb == ModSts.ActiveState]
  (junction 26) -> (junction 32) [order 2]
  (junction 28) -> (junction 142) : {%此接口为接入JA目标类型
      | 	temp_AEB_ObjSty = uint8(1);
      | }
  (junction 32) -> (junction 34) [order 1] : [JA_pedAEBfullFuncImpArb == ModSts.ActiveState]
  (junction 32) -> (junction 148) [order 2]
  (junction 34) -> (junction 36) : {
      | 	temp_AEB_ObjSty = uint8(2);
      | }
  (junction 148) -> (junction 150) [order 1] : [FCTBFuncImpArb == ModSts.ActiveState]
  (junction 148) -> (junction 38) [order 2] : {
      | 	temp_AEB_ObjSty = uint8(0);
      | }
  (junction 150) -> (junction 152) : {
      | 	temp_AEB_ObjSty = uint8(1);
      | }
  (junction 38) -> (junction 18)
  (junction 152) -> (junction 38)
  (junction 36) -> (junction 152)
  (junction 142) -> (junction 36)
  (junction 24) -> (junction 142)
  (junction 16) -> (junction 24)
  (junction 9) -> (junction 16)
  (junction 18) -> (junction 42) : {
      | 	AEB_ObjSty = temp_AEB_ObjSty;
      | }
  data: AEBTrigger input boolean; AEBPartFuncImpArb input Enum: ModSts; EBAfullFuncImpArb input Enum: ModSts; AEBfullFuncImpArb input Enum: ModSts; pedAEBfullFuncImpArb input Enum: ModSts; cycAEBpartFuncImpArb input Enum: ModSts; cycAEBfullFuncImpArb input Enum: ModSts; JA_AEBfullFuncImpArb input Enum: ModSts; JA_pedAEBfullFuncImpArb input Enum: ModSts; TRUE parameter boolean; AEB_ObjSty output uint8; temp_AEB_ObjSty local Enum: ObjectType size=1 =ObjectType.CAR; FCTBFuncImpArb input

== stateflow chart TosAeb/FcwAebJa_Step_sys/ALG_AEBSystem/ALG_AEBOutput/AEBS_IPHUTInfoCalc/AR_HUD_InfoMap/AEB_LeCycFlow ==
  state TargetSelectFunc [function]
    (default) -> (junction 63)
    (junction 63) -> (junction 75) [order 1] : [VehicleConfigAEBCyc == true&&...
        | latCycCalcInfo(index).latCycObjRawInfo.ObjectID ~= CNobjInvalidID &&...
        | latCycCalcInfo(index).latCycObjRawInfo.ObjectType == ObjectTypeCopy.BICYCLE &&...
        | latCycCalcInfo(index).latCycObjRawInfo.dy >= 0 && ...
        | latCycCalcInfo(index).latCycObjRawInfo.dx > 0 &&...
        | latCycCalcInfo(index).CollisionRiskProb > 0.2]
    (junction 63) -> (junction 230) [order 2]
    (junction 75) -> (junction 213) : {
        | 	tgtIsFind = true;
        | }
    (junction 213) -> (junction 215) [order 1] : [(VehicleConfigAEBCyc == true&&...
        | latCycCalcInfo(tgtIndex).latCycObjRawInfo.ObjectID ~= CNobjInvalidID &&...
        | latCycCalcInfo(tgtIndex).latCycObjRawInfo.ObjectType == ObjectTypeCopy.BICYCLE &&...
        | latCycCalcInfo(tgtIndex).latCycObjRawInfo.dy >= 0 && ...
        | latCycCalcInfo(tgtIndex).latCycObjRawInfo.dx > 0 &&...
        | latCycCalcInfo(index).CollisionRiskProb > 0.2) == false]
    (junction 213) -> (junction 128) [order 2] : {
        | 	temp1= latCycCalcInfo(index).latCycObjRawInfo.dx;
        | 	temp2 = latCycCalcInfo(tgtIndex).latCycObjRawInfo.dx;
        | }
    (junction 215) -> (junction 218) : {
        | 	tgtIndex = index;
        | }
    (junction 128) -> (junction 131) [order 1] : [temp1 < temp2]
    (junction 128) -> (junction 101) [order 2]
    (junction 131) -> (junction 133) : {
        | 	tgtIndex = index;
        | }
    (junction 101) -> (junction 230)
    (junction 133) -> (junction 101)
    (junction 218) -> (junction 133)
    (junction 230) -> (junction 233)
  note: AEB_LePedDetn
      |
      | AEB_LePedTyp
      |
      | AEB_LePedHeading
      |
      | AEB_LePedDx
      |
      | AEB_LePedDy
      |
      | AEB_LePed_collisionprob
  (default) -> (junction 112) : {
      | 	tgtIndex = 1;
      | 	tgtIsFind = false;
      |       index = uint8(1);
      | }
  (junction 112) -> (junction 110) [order 1] : [index <= 10]
  (junction 169) -> (junction 112)
  (junction 110) -> (junction 113) : {
      | 	TargetSelectFunc();
      | }
  (junction 112) -> (junction 114) [order 2]
  (junction 113) -> (junction 169) : {
      | 	index = index + 1;
      | }
  (junction 114) -> (junction 172) [order 1] : [tgtIsFind == true]
  (junction 114) -> (junction 176) [order 2] : {
      | 	tgtObj = latCycObjCalcInfoDefaultParams;
      | }
  (junction 172) -> (junction 174) : {
      | 	tgtObj = latCycCalcInfo(tgtIndex);
      | }
  (junction 174) -> (junction 176)
  (junction 176) -> (junction 179)
  (junction 179) -> (junction 235) [order 1] : [tgtObj.latCycObjRawInfo.ObjectID ~= CNobjInvalidID]
  (junction 179) -> (junction 240) [order 2] : {
      |   AEB_LeCycDetn = false;
      |   AEB_LeCycTyp = uint8(0);
      |   AEB_LeCycHeading = single(0);
      |   AEB_LeCycDx = single(0);
      |   AEB_LeCycDy = single(0);
      |   AEB_LeCyc_collisionprob = false;
      | }
  (junction 235) -> (junction 237) : {
      |   AEB_LeCycDetn = true;
      |   AEB_LeCycTyp = uint8(4);
      |   AEB_LeCycHeading = tgtObj.latCycObjRawInfo.ObjectAzimuthAngle;
      |   AEB_LeCycDx = max((tgtObj.latCycObjRawInfo.dx - P_Global_WheelBase_m),0);
      |   AEB_LeCycDy = -tgtObj.latCycObjRawInfo.dy;
      |   AEB_LeCyc_collisionprob = AEB_Cyc_collisionprob;
      | }
  (junction 237) -> (junction 240)
  (junction 240) -> (junction 243)
  data: latCycCalcInfo input Bus: BusLatCycObjCalcInfo size=10; VehicleConfigAEBCyc input; index local uint8; temp1 local; temp2 local; latCycObjCalcInfoDefaultParams parameter Bus: BusLatCycObjCalcInfo; CNobjInvalidID parameter; AEB_LeCycDetn output; AEB_LeCycTyp output; AEB_LeCycHeading output; AEB_LeCycDx output; AEB_LeCycDy output; AEB_LeCyc_collisionprob output; tgtIsFind local; tgtObj local Bus: BusLatCycObjCalcInfo; tgtIndex local uint8; AEB_Cyc_collisionprob input; P_Global_WheelBase_m parameter

== matlab function TosAeb/FcwAebJa_Step_sys/ALG_AEBSystem/ALG_AEBController/AEB_LatObjInfoCalcApp/LatPedObjCalc/Subsystem/latPedObjInfoCalc/ObjColiisionRiskProbCalc/CollisionRiskProbCalc/MATLAB Function ==
  data: dxReginEgoRUCorr input; dyReginEgoRUCorr input; dyReginEgoLLCorr input; dxReginEgoLLCorr input; dxReginObjRUCorr input; dyReginObjRUCorr input; dxReginObjLLCorr input; dyReginObjLLCorr input; collisionRiskProb input; dyCollisionRiskProb input; dxCollisionRiskProb input
function OverlapPlot(dxReginEgoRUCorr, dyReginEgoRUCorr, dyReginEgoLLCorr, dxReginEgoLLCorr,...
    dxReginObjRUCorr, dyReginObjRUCorr, dxReginObjLLCorr, dyReginObjLLCorr,...
    collisionRiskProb, dyCollisionRiskProb, dxCollisionRiskProb)
coder.extrinsic('plot')
coder.extrinsic('num2str')
dxReginEgoLUCorr = dxReginEgoRUCorr;
dyReginEgoLUCorr = dyReginEgoLLCorr;
dxReginEgoRLCorr = dxReginEgoLLCorr;
dyReginEgoRLCorr = dyReginEgoRUCorr;

dxReginObjLUCorr = dxReginObjRUCorr;
dyReginObjLUCorr = dyReginObjLLCorr;
dxReginObjRLCorr = dxReginObjLLCorr;
dyReginObjRLCorr = dyReginObjRUCorr;

xEgo = [dxReginEgoRUCorr,dxReginEgoLUCorr,dxReginEgoLLCorr,dxReginEgoRLCorr];
yEgo = [dyReginEgoRUCorr,dyReginEgoLUCorr,dyReginEgoLLCorr,dyReginEgoRLCorr];
xObj = [dxReginObjRUCorr,dxReginObjLUCorr,dxReginObjLLCorr,dxReginObjRLCorr];
yObj = [dyReginObjRUCorr,dyReginObjLUCorr,dyReginObjLLCorr,dyReginObjRLCorr];

xEgo1 = yEgo;
yEgo1 = -xEgo;
xObj1 = yObj;
yObj1 = -xObj;

plot(xEgo1,yEgo1,'-o',...
    'LineWidth',1','FaceColor','red','EdgeColor','red','FaceAlpha',.2)
hold on
plot(xObj1,yObj1,'-o',...
    'LineWidth',1','FaceColor','blue','EdgeColor','blue','FaceAlpha',.4)

txtEgoRU = ['(',num2str(xEgo1(1)),',',num2str(yEgo1(1)),')'];
text(xEgo1(1),yEgo1(1),txtEgoRU,'Color','red','FontSize',14)
txtEgoLL = ['(',num2str(xEgo1(3)),',',num2str(yEgo1(3)),')'];
text(xEgo1(1),yEgo1(1),txtEgoLL,'Color','red','FontSize',14)

%     text(sum(xEgo1)/4,sum(xEgo1)/4,['dyOverlap is 1 and dxOverlap is 0'])
%     pause(1);

== stateflow chart TosAeb/FcwAebJa_Step_sys/ALG_AEBSystem/ALG_AEBOutput/AEBS_IPHUTInfoCalc/AR_HUD_InfoMap/AEB_LePedFlow ==
  state TargetSelectFunc [function]
    (default) -> (junction 63)
    (junction 63) -> (junction 75) [order 1] : [VehicleConfigAEBPed == true &&...
        | latPedCalcInfo(index).latPedObjRawInfo.ObjectID ~= CNobjInvalidID &&...
        | latPedCalcInfo(index).latPedObjRawInfo.ObjectType == ObjectTypeCopy.PEDESTRIAN &&...
        | latPedCalcInfo(index).latPedObjRawInfo.dy >= 0 && ...
        | latPedCalcInfo(index).latPedObjRawInfo.dx > 0 &&...
        | latPedCalcInfo(index).CollisionRiskProb > 0.2]
    (junction 63) -> (junction 230) [order 2]
    (junction 75) -> (junction 213) : {
        | 	tgtIsFind = true;
        | }
    (junction 213) -> (junction 215) [order 1] : [(VehicleConfigAEBPed == true &&...
        | latPedCalcInfo(tgtIndex).latPedObjRawInfo.ObjectID ~= CNobjInvalidID &&...
        | latPedCalcInfo(tgtIndex).latPedObjRawInfo.ObjectType == ObjectTypeCopy.PEDESTRIAN &&...
        | latPedCalcInfo(tgtIndex).latPedObjRawInfo.dy >= 0 && ...
        | latPedCalcInfo(tgtIndex).latPedObjRawInfo.dx > 0 &&...
        | latPedCalcInfo(index).CollisionRiskProb > 0.2) == false]
    (junction 213) -> (junction 128) [order 2] : {
        | 	temp1= latPedCalcInfo(index).latPedObjRawInfo.dx;
        | 	temp2 = latPedCalcInfo(tgtIndex).latPedObjRawInfo.dx;
        | }
    (junction 215) -> (junction 218) : {
        | 	tgtIndex = index;
        | }
    (junction 128) -> (junction 131) [order 1] : [temp1 < temp2]
    (junction 128) -> (junction 101) [order 2]
    (junction 131) -> (junction 133) : {
        | 	tgtIndex = index;
        | }
    (junction 101) -> (junction 230)
    (junction 133) -> (junction 101)
    (junction 218) -> (junction 133)
    (junction 230) -> (junction 233)
  note: AEB_LePedDetn
      |
      | AEB_LePedTyp
      |
      | AEB_LePedHeading
      |
      | AEB_LePedDx
      |
      | AEB_LePedDy
      |
      | AEB_LePed_collisionprob
  (default) -> (junction 112) : {
      | 	tgtIndex = 1;
      | 	tgtIsFind = false;
      |       index = uint8(1);
      | }
  (junction 112) -> (junction 110) [order 1] : [index <= 10]
  (junction 169) -> (junction 112)
  (junction 110) -> (junction 113) : {
      | 	TargetSelectFunc();
      | }
  (junction 112) -> (junction 114) [order 2]
  (junction 113) -> (junction 169) : {
      | 	index = index + 1;
      | }
  (junction 114) -> (junction 172) [order 1] : [tgtIsFind == true]
  (junction 114) -> (junction 176) [order 2] : {
      | 	tgtObj = latPedObjCalcInfoDefaultParams;
      | }
  (junction 172) -> (junction 174) : {
      | 	tgtObj = latPedCalcInfo(tgtIndex);
      | }
  (junction 174) -> (junction 176)
  (junction 176) -> (junction 179)
  (junction 179) -> (junction 235) [order 1] : [tgtObj.latPedObjRawInfo.ObjectID ~= CNobjInvalidID]
  (junction 179) -> (junction 240) [order 2] : {
      |   AEB_LePedDetn = false;
      |   AEB_LePedTyp = uint8(0);
      |   AEB_LePedHeading = single(0);
      |   AEB_LePedDx = single(0);
      |   AEB_LePedDy = single(0);
      |   AEB_LePed_collisionprob = false;
      | }
  (junction 235) -> (junction 237) : {
      |   AEB_LePedDetn = true;
      |   AEB_LePedTyp = uint8(4);
      |   AEB_LePedHeading = tgtObj.latPedObjRawInfo.ObjectAzimuthAngle;
      |   AEB_LePedDx = max((tgtObj.latPedObjRawInfo.dx - P_Global_WheelBase_m),0);
      |   AEB_LePedDy = -tgtObj.latPedObjRawInfo.dy;
      |   AEB_LePed_collisionprob = AEB_Ped_collisionprob;
      | }
  (junction 237) -> (junction 240)
  (junction 240) -> (junction 243)
  data: latPedCalcInfo input Bus: BusLatPedObjCalcInfo size=10; VehicleConfigAEBPed input; index local uint8; temp1 local; temp2 local; latPedObjCalcInfoDefaultParams parameter Bus: BusLatPedObjCalcInfo; CNobjInvalidID parameter; AEB_LePedDetn output; AEB_LePedTyp output; AEB_LePedHeading output; AEB_LePedDx output; AEB_LePedDy output; AEB_LePed_collisionprob output; tgtIndex local uint8; tgtObj local Bus: BusLatPedObjCalcInfo; tgtIsFind local; AEB_Ped_collisionprob input; P_Global_WheelBase_m parameter

== stateflow chart TosAeb/FcwAebJa_Step_sys/ALG_AEBSystem/ALG_AEBOutput/AEBS_IPHUTInfoCalc/AR_HUD_InfoMap/AEB_RiCycFlow ==
  state TargetSelectFunc [function]
    (default) -> (junction 63)
    (junction 63) -> (junction 75) [order 1] : [VehicleConfigAEBCyc == true&&...
        | latCycCalcInfo(index).latCycObjRawInfo.ObjectID ~= CNobjInvalidID &&...
        | latCycCalcInfo(index).latCycObjRawInfo.ObjectType == ObjectTypeCopy.BICYCLE &&...
        | latCycCalcInfo(index).latCycObjRawInfo.dy < 0 && ...
        | latCycCalcInfo(index).latCycObjRawInfo.dx > 0 &&...
        | latCycCalcInfo(index).CollisionRiskProb > 0.2]
    (junction 63) -> (junction 230) [order 2]
    (junction 75) -> (junction 213) : {
        | 	tgtIsFind = true;
        | }
    (junction 213) -> (junction 215) [order 1] : [(VehicleConfigAEBCyc == true&&...
        | latCycCalcInfo(tgtIndex).latCycObjRawInfo.ObjectID ~= CNobjInvalidID &&...
        | latCycCalcInfo(tgtIndex).latCycObjRawInfo.ObjectType == ObjectTypeCopy.BICYCLE &&...
        | latCycCalcInfo(tgtIndex).latCycObjRawInfo.dy < 0 && ...
        | latCycCalcInfo(tgtIndex).latCycObjRawInfo.dx > 0 &&...
        | latCycCalcInfo(index).CollisionRiskProb > 0.2) == false]
    (junction 213) -> (junction 128) [order 2] : {
        | 	temp1= latCycCalcInfo(index).latCycObjRawInfo.dx;
        | 	temp2 = latCycCalcInfo(tgtIndex).latCycObjRawInfo.dx;
        | }
    (junction 215) -> (junction 218) : {
        | 	tgtIndex = index;
        | }
    (junction 128) -> (junction 131) [order 1] : [temp1 < temp2]
    (junction 128) -> (junction 101) [order 2]
    (junction 131) -> (junction 133) : {
        | 	tgtIndex = index;
        | }
    (junction 101) -> (junction 230)
    (junction 133) -> (junction 101)
    (junction 218) -> (junction 133)
    (junction 230) -> (junction 233)
  note: AEB_LePedDetn
      |
      | AEB_LePedTyp
      |
      | AEB_LePedHeading
      |
      | AEB_LePedDx
      |
      | AEB_LePedDy
      |
      | AEB_LePed_collisionprob
  (default) -> (junction 112) : {
      | 	tgtIndex = 1;
      | 	tgtIsFind = false;
      |       index = uint8(1);
      | }
  (junction 112) -> (junction 110) [order 1] : [index <= 10]
  (junction 169) -> (junction 112)
  (junction 110) -> (junction 113) : {
      | 	TargetSelectFunc();
      | }
  (junction 112) -> (junction 114) [order 2]
  (junction 113) -> (junction 169) : {
      | 	index = index + 1;
      | }
  (junction 114) -> (junction 172) [order 1] : [tgtIsFind == true]
  (junction 114) -> (junction 176) [order 2] : {
      | 	tgtObj = latCycObjCalcInfoDefaultParams;
      | }
  (junction 172) -> (junction 174) : {
      | 	tgtObj = latCycCalcInfo(tgtIndex);
      | }
  (junction 174) -> (junction 176)
  (junction 176) -> (junction 179)
  (junction 179) -> (junction 235) [order 1] : [tgtObj.latCycObjRawInfo.ObjectID ~= CNobjInvalidID]
  (junction 179) -> (junction 240) [order 2] : {
      |   AEB_RiCycDetn = false;
      |   AEB_RiCycTyp = uint8(0);
      |   AEB_RiCycHeading = single(0);
      |   AEB_RiCycDx = single(0);
      |   AEB_RiCycDy = single(0);
      |   AEB_RiCyc_collisionprob = false;
      | }
  (junction 235) -> (junction 237) : {
      |   AEB_RiCycDetn = true;
      |   AEB_RiCycTyp = uint8(4);
      |   AEB_RiCycHeading = tgtObj.latCycObjRawInfo.ObjectAzimuthAngle;
      |   AEB_RiCycDx = max((tgtObj.latCycObjRawInfo.dx - P_Global_WheelBase_m),0);
      |   AEB_RiCycDy = -tgtObj.latCycObjRawInfo.dy;
      |   AEB_RiCyc_collisionprob = AEB_Cyc_collisionprob;
      | }
  (junction 237) -> (junction 240)
  (junction 240) -> (junction 243)
  data: AEB_Cyc_collisionprob input; VehicleConfigAEBCyc input; latCycCalcInfo input Bus: BusLatCycObjCalcInfo size=10; index local uint8; temp1 local; temp2 local; latCycObjCalcInfoDefaultParams parameter Bus: BusLatCycObjCalcInfo; CNobjInvalidID parameter; AEB_RiCycDetn output; AEB_RiCycTyp output; AEB_RiCycHeading output; AEB_RiCycDx output; AEB_RiCycDy output; AEB_RiCyc_collisionprob output; tgtIsFind local; tgtObj local Bus: BusLatCycObjCalcInfo; tgtIndex local uint8; P_Global_WheelBase_m parameter
